simulink model "MX_Gateway"  (Simulink 9.0) (continued)
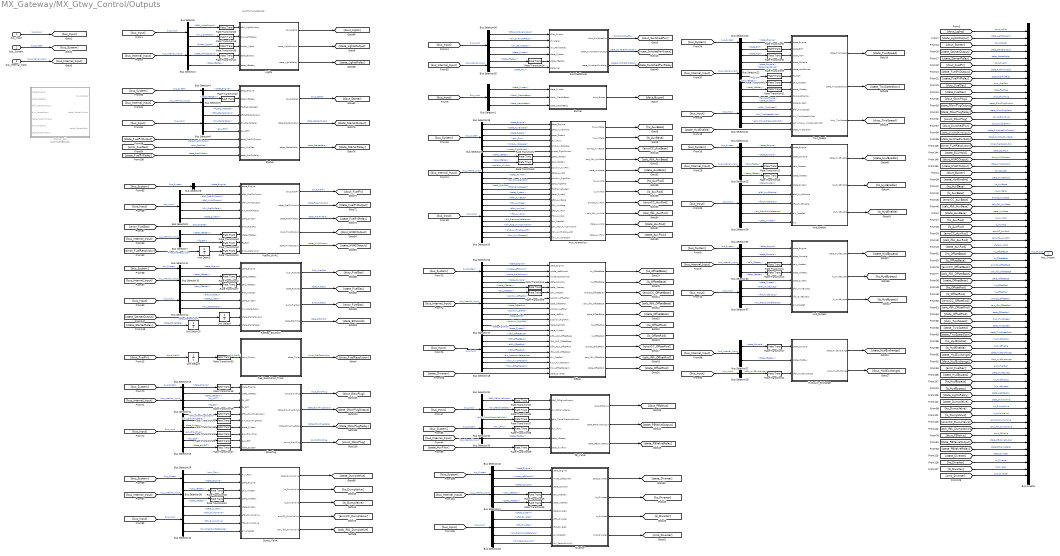
== system MX_Gateway/MX_Gtwy_Control/Outputs ==
  note: OUTPUT HANDLING
  bus_Input : Inport
  bus_System : Inport  Port=2
  bus_Internal_Input : Inport  Port=3
  Aux_Hydraulics : Subsystem  TreatAsAtomicUnit=on  SystemSampleTime=srate  RTWFileName=mod_Outputs
      settings: RTWSystemCode=Nonreusable function  RTWFcnNameOpts=Use subsystem name  RTWFileNameOpts=User specified
  Bus Creator : BusCreator  Inputs=67
  Bus Selector : BusSelector  OutputSignals=bus_DigitalInputs.dfbk_LightsOutput,bus_DigitalInputs.din_LightsRelay
  Bus Selector1 : BusSelector  OutputSignals=bus_Controller.state_System,bus_CAN.bus_Secondary.delay_DepthBeep,bus_CAN.bus_Secondary.state_DepthBeep
  Bus Selector10 : BusSelector  OutputSignals=state_VBattery,calc_VBattery
  Bus Selector{4,8,11,13,23..24,33} (7 alike) : BusSelector  OutputSignals=state_Engine
  Bus Selector12 : BusSelector  OutputSignals=bus_AnalogFdbk.afbk_FuelSec,bus_Controller.state_System,bus_DigitalInputs.din_StarterRelay
  Bus Selector14 : BusSelector  OutputSignals=state_VBattery,flt_HOT,flt_ECT
  Bus Selector15 : BusSelector  OutputSignals=bus_DigitalInputs.dfbk_GlowPlugOutput,bus_DigitalInputs.din_GlowPlugRelay,bus_Controller.state_System,bus_CAN.bus_ECU.ecu_GlowPlug,bus_CAN.bus_ECU.ecu_ECT,bus_CAN.bus_ECU.state_ecuECT
  Bus Selector16 : BusSelector  OutputSignals=state_Engine,mode_LeftControl,mode_RightControl
  Bus Selector17 : BusSelector  OutputSignals=state_Console,state_VBattery,calc_VBattery,position_OffsetPot,state_OffsetPot,position_LeftPot,state_LeftPot,position_RightPot,state_RightPot
  Bus Selector18 : BusSelector  OutputSignals=bus_Controller.state_System,bus_AnalogFdbk.afbk_OffsetBase,bus_CurrentFdbk.ifbk_OffsetBase,bus_AnalogFdbk.afbk_OffsetRod,bus_CurrentFdbk.ifbk_OffsetRod,bus_AnalogInputs.ain_PrecisionReference,bus_CAN.bus_DeluxeG5.flow_Aux2Percent,bus_CAN.bus_DeluxeG5.flow_OffsetPercent
  Bus Selector19 : BusSelector  OutputSignals=state_Engine,state_AuxEnable,state_Detent,aux_Flow,cmd_AuxRelease,mode_RightControl
  Bus Selector2 : BusSelector  OutputSignals=button_Lights,state_PanelButtons,state_VBattery
  Bus Selector20 : BusSelector  OutputSignals=bus_Controller.state_System,bus_AnalogFdbk.afbk_AuxBase,bus_CurrentFdbk.ifbk_AuxBase,bus_AnalogFdbk.afbk_AuxRod,bus_CurrentFdbk.ifbk_AuxRod,bus_AnalogInputs.ain_PrecisionReference,bus_CAN.bus_ACD.can_ACD_AuxCommand,bus_CAN.bus_ACD.cmd_AuxEnable_ACD,bus_CAN.bus_DeluxeG5.flow_Aux1Percent
  Bus Selector21 : BusSelector  OutputSignals=bus_Controller.state_System,bus_AnalogFdbk.afbk_TwoSpeed,bus_DigitalInputs.din_TwoSpeedSwitch,bus_CombinedInputs.adin_TravelPressureSwitch
  Bus Selector22 : BusSelector  OutputSignals=state_ECT,state_HOT,state_Console,state_LoadSense,flt_HOT,calc_VBattery,state_VBattery,bar_LoadSense
  Bus Selector25 : BusSelector  OutputSignals=state_Console,calc_VBattery,state_VBattery
  Bus Selector26 : BusSelector  OutputSignals=bus_Controller.state_System,bus_AnalogFdbk.afbk_HydEnable,bus_CurrentFdbk.ifbk_HydEnable,bus_AnalogInputs.ain_PrecisionReference,CANOpenInMsg.state_Inverter
  Bus Selector27 : BusSelector  OutputSignals=state_Console,state_VBattery
  Bus Selector28 : BusSelector  OutputSignals=bus_DigitalInputs.dfbk_HydExchange
  Bus Selector29 : BusSelector  OutputSignals=aux_Flow,state_Engine
  Bus Selector3 : BusSelector  OutputSignals=state_VBattery,calc_VBattery,position_AuxPot,state_AuxPot,position_RightPot,state_RightPot,flt_EngineSpeed
  Bus Selector30 : BusSelector  OutputSignals=state_VBattery
  Bus Selector31 : BusSelector  OutputSignals=bus_DigitalInputs.dfbk_PBValveOutput,bus_DigitalInputs.din_PBValveRelay,bus_CombinedInputs.adin_TravelPressureSwitch
  Bus Selector32 : BusSelector  OutputSignals=aux_Flow
  Bus Selector34 : BusSelector  OutputSignals=state_Console,calc_VBattery,state_VBattery
  Bus Selector35 : BusSelector  OutputSignals=state_VBattery,state_Key
  Bus Selector36 : BusSelector  OutputSignals=bus_DigitalInputs.dfbk_SwPowerOutput,bus_DigitalInputs.din_SwPowerRelay,bus_DigitalInputs.din_RemoteKey,bus_Controller.state_System
  Bus Selector37 : BusSelector  OutputSignals=bus_Controller.state_System,bus_AnalogFdbk.afbk_HydBypass,bus_CurrentFdbk.ifbk_HydBypass,bus_AnalogInputs.ain_PrecisionReference
  Bus Selector38 : BusSelector  OutputSignals=calc_VBattery,state_VBattery
  Bus Selector42 : BusSelector  OutputSignals=bus_Controller.state_System,bus_AnalogFdbk.afbk_DumpValve,bus_CurrentFdbk.ifbk_DumpValve,bus_AnalogInputs.ain_PrecisionReference
  Bus Selector46 : BusSelector  OutputSignals=state_Engine,mode_LeftControl
  Bus Selector47 : BusSelector  OutputSignals=state_Console,calc_VBattery,state_VBattery
  Bus Selector48 : BusSelector  OutputSignals=bus_Controller.state_System,bus_AnalogFdbk.afbk_Diverter,bus_CurrentFdbk.ifbk_Diverter,bus_AnalogInputs.ain_PrecisionReference,bus_DigitalInputs.din_SecondAuxSw
  Bus Selector5 : BusSelector  OutputSignals=state_VBattery,flt_EngineSpeed
  Bus Selector6 : BusSelector  OutputSignals=bus_DigitalInputs.dfbk_StarterOutput,bus_DigitalInputs.din_StarterRelay,bus_CAN.bus_ECU.ecu_EOT
  Bus Selector7 : BusSelector  OutputSignals=state_VBattery,flt_ECT
  Bus Selector9 : BusSelector  OutputSignals=bus_DigitalInputs.dfbk_FuelPriOutput,bus_DigitalInputs.dfbk_HVACOutput,bus_DigitalInputs.din_FuelPriRelay,bus_Controller.state_System
  Buzzer : Subsystem  TreatAsAtomicUnit=on  SystemSampleTime=srate  RTWFileName=mod_Outputs
      settings: RTWSystemCode=Nonreusable function  RTWFileNameOpts=User specified
  Diverter : Subsystem  TreatAsAtomicUnit=on  SystemSampleTime=srate  RTWFileName=mod_Outputs
      settings: RTWSystemCode=Nonreusable function  RTWFcnNameOpts=Use subsystem name  RTWFileNameOpts=User specified
  Dump_Valve : Subsystem  TreatAsAtomicUnit=on  SystemSampleTime=srate  RTWFileName=mod_Outputs
      settings: RTWSystemCode=Nonreusable function  RTWFcnNameOpts=Use subsystem name  RTWFileNameOpts=User specified
  FuelPri_HVAC : Subsystem  TreatAsAtomicUnit=on  SystemSampleTime=srate  RTWFileName=mod_Outputs
      settings: RTWSystemCode=Nonreusable function  RTWFileNameOpts=User specified
  FuelSec_ECUHold : Subsystem  TreatAsAtomicUnit=on  SystemSampleTime=srate  RTWFileName=mod_Outputs
      settings: RTWSystemCode=Nonreusable function  RTWFileNameOpts=User specified
  Fuel_Resolution_Timer : Subsystem  TreatAsAtomicUnit=on  SystemSampleTime=srate100  RTWFileName=mod_Outputs  mask{CD_PRIMARY_FUNCTION=CD_FUEL_PRIMARY_FUNCTION, CD_RESOLUTION_TIMER=CD_FUEL_RESOLUTION_TIMER}
      mask prompts: CD_PRIMARY_FUNCTION "Primary Function"; CD_RESOLUTION_TIMER "Resolution Timer"
      settings: RTWSystemCode=Nonreusable function  RTWFcnNameOpts=Use subsystem name  RTWFileNameOpts=User specified
  GlowPlug : Subsystem  TreatAsAtomicUnit=on  SystemSampleTime=srate100  RTWFileName=mod_Outputs
      settings: RTWSystemCode=Nonreusable function  RTWFileNameOpts=User specified
  Hyd_Bypass : Subsystem  TreatAsAtomicUnit=on  SystemSampleTime=srate  RTWFileName=mod_Outputs
      settings: RTWSystemCode=Nonreusable function  RTWFcnNameOpts=Use subsystem name  RTWFileNameOpts=User specified
  Hyd_Enable : Subsystem  TreatAsAtomicUnit=on  SystemSampleTime=srate  RTWFileName=mod_Outputs
      settings: RTWSystemCode=Nonreusable function  RTWFcnNameOpts=Use subsystem name  RTWFileNameOpts=User specified
  Hydraulic_Exchange : Subsystem  TreatAsAtomicUnit=on  SystemSampleTime=srate100  RTWFileName=mod_Outputs
      settings: RTWSystemCode=Nonreusable function  RTWFcnNameOpts=Use subsystem name  RTWFileNameOpts=User specified
  Lights : Subsystem  TreatAsAtomicUnit=on  SystemSampleTime=srate100  RTWFileName=mod_Outputs
      settings: RTWSystemCode=Nonreusable function  RTWFileNameOpts=User specified
  Offset : Subsystem  TreatAsAtomicUnit=on  SystemSampleTime=srate  RTWFileName=mod_Outputs
      settings: RTWSystemCode=Nonreusable function  RTWFcnNameOpts=Use subsystem name  RTWFileNameOpts=User specified
  PB_Valve : Subsystem  TreatAsAtomicUnit=on  SystemSampleTime=srate100  RTWFileName=mod_Outputs
      settings: RTWSystemCode=Nonreusable function  RTWFileNameOpts=User specified
  Rate Transition{1..18,20..22,24..42,44..46} (43 alike) : RateTransition  Integrity=off
  Starter : Subsystem  TreatAsAtomicUnit=on  SystemSampleTime=srate  RTWFileName=mod_Outputs
      settings: RTWSystemCode=Nonreusable function  RTWFileNameOpts=User specified
  Starter_old : Subsystem  Commented=on  TreatAsAtomicUnit=on  SystemSampleTime=srate  RTWFileName=mod_Outputs
      settings: RTWSystemCode=Nonreusable function  RTWFileNameOpts=User specified
  SwitchedPower : Subsystem  TreatAsAtomicUnit=on  SystemSampleTime=srate  RTWFileName=mod_Outputs
      settings: RTWSystemCode=Nonreusable function  RTWFileNameOpts=User specified
  Two_Speed : Subsystem  TreatAsAtomicUnit=on  SystemSampleTime=srate  RTWFileName=mod_Outputs
      settings: RTWSystemCode=Nonreusable function  RTWFcnNameOpts=Use subsystem name  RTWFileNameOpts=User specified
  Unit Delay{3,5,9..10} (4 alike) : UnitDelay  SampleTime=-1
      settings: AttributesFormatString=<initial=%<X0>>\n<tsample=%<SampleTime>>
  bus_Output : Outport
  wires:
  Lights/1(dout_Lights) -> [dout_Lights]
  Bus Selector4/1 -> Starter/1(state_Engine)  "<state_Engine>"
  Starter/1(dout_Starter) -> [dout_Starter]  "dout_Starter"
  FuelPri_HVAC/1(dout_FuelPri) -> [dout_FuelPri]  "dout_FuelPri"
  FuelPri_HVAC/3(state_FuelPriRelay) -> [state_FuelPriRelay]  "state_FuelPriRelay"
  FuelPri_HVAC/2(state_FuelPriOutput) -> [state_FuelPriOutput]  "state_FuelPriOutput"
  Starter/2(state_StarterOutput) -> [state_StarterOutput]  "state_StarterOutput"
  Starter/3(state_StarterRelay) -> [state_StarterRelay]  "state_StarterRelay"
  Lights/2(state_LightsOutput) -> [state_LightsOutput]
  [error_FuelSec] -> Starter/8(error_FuelSec)  "error_FuelSec"
  Bus Selector14/3 -> GlowPlug/4(flt_ECT)  "<flt_ECT>"
  Bus Selector14/2 -> GlowPlug/3(flt_HOT)  "<flt_HOT>"
  Bus Selector14/1 -> GlowPlug/2(state_VBattery)  "<state_VBattery>"
  GlowPlug/1(dout_GlowPlug) -> [dout_GlowPlug]  "dout_GlowPlug"
  GlowPlug/2(state_GlowPlugOutput) -> [state_GlowPlugOutput]  "state_GlowPlugOutput"
  GlowPlug/3(state_GlowPlugRelay) -> [state_GlowPlugRelay]  "state_GlowPlugRelay"
  FuelSec_ECUHold/1(duty_FuelSec) -> [duty_FuelSec]  "duty_FuelSec"
  FuelSec_ECUHold/2(state_FuelSec) -> [state_FuelSec]  "state_FuelSec"
  [state_FuelPriRelay] -> Starter/9(state_FuelPriRelay)  "state_FuelPriRelay"
  GlowPlug/4(count_GlowPlug) -> [count_GlowPlug]  "count_GlowPlug"
  Rate Transition16/1 -> GlowPlug/1(state_Engine)
  SwitchedPower/1(dout_SwitchedPwr) -> [dout_SwitchedPwr]  "dout_SwitchedPwr"
  SwitchedPower/2(state_SwitchedPwrOutput) -> [state_SwitchedPwrOutput]  "state_SwitchedPwrOutput"
  SwitchedPower/3(state_SwitchedPwrRelay) -> [state_SwitchedPwrRelay]  "state_SwitchedPwrRelay"
  Bus Selector36/1 -> SwitchedPower/1(dkbk_Output)  "<dfbk_SwPowerOutput>"
  Bus Selector36/2 -> SwitchedPower/2(din_Relay)  "<din_SwPowerRelay>"
  Bus Selector35/1 -> Rate Transition14/1  "<state_VBattery>"
  Bus Selector35/2 -> SwitchedPower/6(state_Key)  "<state_Key>"
  bus_Input/1 -> [bus_Input]  "bus_Input"
  bus_System/1 -> [bus_System]  "bus_System"
  bus_Internal_Input/1 -> [bus_Internal_Input]  "bus_Internal_Input"
  [bus_Input] -> Bus Selector/1  "bus_Input"
  [bus_Internal_Input] -> Bus Selector2/1  "bus_Internal_Inputs"
  [dout_Lights] -> Bus Creator/1  "dout_Lights"
  [state_LightsOutput] -> Bus Creator/2  "state_LightsOutput"
  [dout_Starter] -> Bus Creator/3  "dout_Starter"
  [state_StarterOutput] -> Bus Creator/4  "state_StarterOutput"
  [state_StarterRelay] -> Bus Creator/5  "state_StarterRelay"
  [dout_FuelPri] -> Bus Creator/6  "dout_FuelPri"
  [state_FuelPriOutput] -> Bus Creator/7  "state_FuelPriOutput"
  [state_FuelPriRelay] -> Bus Creator/8  "state_FuelPriRelay"
  [duty_FuelSec] -> Bus Creator/9  "duty_FuelSec"
  [state_FuelSec] -> Bus Creator/10  "state_FuelSec"
  [dout_GlowPlug] -> Bus Creator/11  "dout_GlowPlug"
  [state_GlowPlugOutput] -> Bus Creator/12  "state_GlowPlugOutput"
  [state_GlowPlugRelay] -> Bus Creator/13  "state_GlowPlugRelay"
  [count_GlowPlug] -> Bus Creator/14  "count_GlowPlug"
  [dout_SwitchedPwr] -> Bus Creator/15  "dout_SwitchedPwr"
  [state_SwitchedPwrOutput] -> Bus Creator/16  "state_SwitchedPwrOutput"
  [state_SwitchedPwrRelay] -> Bus Creator/17  "state_SwitchedPwrRelay"
  Bus Selector2/3 -> Lights/5(state_VBattery)  "<state_VBattery>"
  [bus_System] -> Bus Selector4/1  "bus_System"
  [state_FuelPriOutput] -> Starter/7(state_FuelPriOutput)  "state_FuelPriOutput"
  [bus_Internal_Input] -> Bus Selector5/1  "bus_Internal_Inputs"
  Bus Selector5/1 -> Rate Transition20/1  "<state_VBattery>"
  [bus_Input] -> Bus Selector6/1  "bus_Input"
  Bus Selector6/1 -> Starter/4(dfbk_StarterOutput)  "<dfbk_StarterOutput>"
  Bus Selector6/2 -> Starter/5(din_StarterRelay)  "<din_StarterRelay>"
  [bus_Internal_Input] -> Bus Selector7/1  "bus_Internal_Inputs"
  Bus Selector8/1 -> FuelPri_HVAC/1(state_Engine)  "<state_Engine>"
  [bus_System] -> Bus Selector8/1  "bus_System"
  [bus_Input] -> Bus Selector9/1  "bus_Input"
  Bus Selector9/1 -> FuelPri_HVAC/2(dfbk_FuelPriOutput)  "<dfbk_FuelPriOutput>"
  Bus Selector9/3 -> FuelPri_HVAC/4(din_FuelPriRelay)  "<din_FuelPriRelay>"
  Bus Selector7/1 -> Rate Transition34/1  "<state_VBattery>"
  [bus_Internal_Input] -> Bus Selector10/1  "bus_Internal_Inputs"
  Bus Selector11/1 -> FuelSec_ECUHold/1(state_Engine)  "<state_Engine>"
  [bus_System] -> Bus Selector11/1  "bus_System"
  [bus_Input] -> Bus Selector12/1  "bus_Input"
  Bus Selector13/1 -> Rate Transition16/1  "<state_Engine>"
  [bus_System] -> Bus Selector13/1  "bus_System"
  [bus_Internal_Input] -> Bus Selector14/1  "bus_Internal_Input"
  [bus_Input] -> Bus Selector15/1  "bus_Input"
  Bus Selector15/1 -> Rate Transition29/1  "<dfbk_GlowPlugOutput>"
  Bus Selector15/2 -> Rate Transition30/1  "<din_GlowPlugRelay>"
  Bus Selector15/3 -> Rate Transition44/1  "<state_System>"
  [bus_System] -> Bus Selector16/1  "bus_System"
  [bus_Internal_Input] -> Bus Selector17/1  "bus_Internal_Input"
  [bus_Input] -> Bus Selector18/1  "bus_Input"
  [bus_Internal_Input] -> Bus Selector35/1  "bus_Internal_Input"
  [bus_Input] -> Bus Selector36/1  "bus_Input"
  Rate Transition20/1 -> Starter/2(state_VBattery)
  [dout_FuelPri] -> Unit Delay3/1  "dout_FuelPri"
  Unit Delay3/1 -> Rate Transition33/1
  Fuel_Resolution_Timer/1(state_Timer) -> [timer_FuelResolution]  "timer_FuelResolution"
  [timer_FuelResolution] -> Unit Delay5/1  "timer_FuelResolution"
  Unit Delay5/1 -> Rate Transition41/1
  Bus Selector12/2 -> FuelSec_ECUHold/5(state_System)  "<state_System>"
  Bus Selector7/2 -> Rate Transition40/1  "<flt_ECT>"
  Rate Transition33/1 -> Fuel_Resolution_Timer/1(dout_Primary)  "dout_FuelPri"
  Bus Selector/1 -> Rate Transition25/1  "<dfbk_LightsOutput>"
  Bus Selector36/3 -> SwitchedPower/3(din_RemoteKey)  "<din_RemoteKey>"
  FuelSec_ECUHold/3(error_FuelSec) -> [error_FuelSec]  "error_FuelSec"
  [timer_FuelResolution] -> Bus Creator/18  "timer_FuelResolution"
  Rate Transition44/1 -> GlowPlug/7(state_System)
  Rate Transition22/1 -> Lights/4(state_Buttons)
  Rate Transition36/1 -> Lights/3(button_lights)
  Bus Selector10/1 -> Rate Transition42/1  "<state_VBattery>"
  Bus Selector10/2 -> Rate Transition7/1  "<calc_VBattery>"
  Bus Selector12/1 -> FuelSec_ECUHold/4(afbk_FuelSec)  "<afbk_FuelSec>"
  Bus Selector36/4 -> SwitchedPower/4(state_System)  "<state_System>"
  FuelSec_ECUHold/4(state_ECUHold) -> [state_ECUHold]  "state_ECUHold"
  [state_ECUHold] -> Bus Creator/19  "state_ECUHold"
  [state_StarterOutput] -> Unit Delay9/1  "state_StarterOutput"
  [state_StarterRelay] -> Unit Delay10/1  "state_StarterRelay"
  Unit Delay9/1 -> FuelSec_ECUHold/7(state_StarterOutput)
  Unit Delay10/1 -> FuelSec_ECUHold/8(state_StarterRelay)
  Bus Selector12/3 -> FuelSec_ECUHold/6(din_StarterRelay)  "<din_StarterRelay>"
  Rate Transition34/1 -> FuelPri_HVAC/7(state_VBattery)
  Rate Transition40/1 -> FuelPri_HVAC/8(flt_ECT)
  Rate Transition41/1 -> FuelPri_HVAC/9(timer_Resolution)
  Rate Transition42/1 -> FuelSec_ECUHold/2(state_VBattery)
  Rate Transition7/1 -> FuelSec_ECUHold/3(calc_VBattery)
  [error_FuelSec] -> FuelPri_HVAC/6(error_FuelSec)  "error_FuelSec"
  FuelPri_HVAC/4(dout_HVACOutput) -> [dout_HVACOutput]
  FuelPri_HVAC/5(state_HVACOutput) -> [state_HVACOutput]  "state_HVACOutput"
  [dout_HVACOutput] -> Bus Creator/20  "dout_HVACOutput"
  [state_HVACOutput] -> Bus Creator/21  "state_HVACOutput"
  Bus Selector9/4 -> FuelPri_HVAC/5(state_System)  "<state_System>"
  Rate Transition14/1 -> SwitchedPower/5(state_VBattery)
  Buzzer/1(dout_Buzzer) -> [dout_Buzzer]
  [dout_Buzzer] -> Bus Creator/22  "dout_Buzzer"
  [bus_Input] -> Bus Selector1/1  "bus_Input"
  Bus Selector1/1 -> Buzzer/1(state_System)  "<state_System>"
  Two_Speed/1(state_TwoSpeed) -> [state_TwoSpeed]
  [state_HydEnable] -> Bus Creator/23  "state_HydEnable"
  [bus_Internal_Input] -> Bus Selector3/1  "bus_Internal_Input"
  [bus_System] -> Bus Selector19/1  "bus_System"
  [bus_Input] -> Bus Selector20/1  "bus_Input"
  Bus Selector19/1 -> Aux_Hydraulics/1(state_Engine)  "<state_Engine>"
  Bus Selector3/1 -> Rate Transition2/1  "<state_VBattery>"
  Bus Selector3/2 -> Rate Transition3/1  "<calc_VBattery>"
  Bus Selector20/1 -> Aux_Hydraulics/14(state_System)  "<state_System>"
  Bus Selector20/2 -> Aux_Hydraulics/15(afbk_AuxBase)  "<afbk_AuxBase>"
  Bus Selector20/3 -> Aux_Hydraulics/16(ifbk_AuxBase)  "<ifbk_AuxBase>"
  Bus Selector20/4 -> Aux_Hydraulics/17(afbk_AuxRod)  "<afbk_AuxRod>"
  Bus Selector20/5 -> Aux_Hydraulics/18(ifbk_AuxRod)  "<ifbk_AuxRod>"
  Aux_Hydraulics/1(hs_AuxBase) -> [hs_AuxBase]
  Aux_Hydraulics/2(ls_AuxBase) -> [ls_AuxBase]
  Aux_Hydraulics/3(errorCC_AuxBase) -> [errorCC_AuxBase]
  Aux_Hydraulics/4(calc_ifbk_AuxBase) -> [calc_ifbk_AuxBase]
  Aux_Hydraulics/5(state_AuxBase) -> [state_AuxBase]
  Aux_Hydraulics/6(hs_AuxRod) -> [hs_AuxRod]
  Aux_Hydraulics/7(ls_AuxRod) -> [ls_AuxRod]
  Aux_Hydraulics/8(errorCC_AuxRod) -> [errorCC_AuxRod]
  Aux_Hydraulics/9(calc_ifbk_AuxRod) -> [calc_ifbk_AuxRod]
  Aux_Hydraulics/10(state_AuxRod) -> [state_AuxRod]
  [hs_AuxBase] -> Bus Creator/24  "hs_AuxBase"
  [errorCC_AuxBase] -> Bus Creator/26  "errorCC_AuxBase"
  [calc_ifbk_AuxBase] -> Bus Creator/27  "calc_ifbk_AuxBase"
  [state_AuxBase] -> Bus Creator/28  "state_AuxBase"
  [hs_AuxRod] -> Bus Creator/29  "hs_AuxRod"
  [ls_AuxRod] -> Bus Creator/30  "ls_AuxRod"
  [errorCC_AuxRod] -> Bus Creator/31  "errorCC_AuxRod"
  [calc_ifbk_AuxRod] -> Bus Creator/32  "calc_ifbk_AuxRod"
  [state_AuxRod] -> Bus Creator/33  "state_AuxRod"
  Bus Selector16/1 -> Offset/1(state_Engine)  "<state_Engine>"
  Bus Selector18/1 -> Offset/13(state_System)  "<state_System>"
  Bus Selector17/1 -> Offset/4(state_Console)  "<state_Console>"
  Bus Selector17/2 -> Rate Transition4/1  "<state_VBattery>"
  Bus Selector17/3 -> Rate Transition5/1  "<calc_VBattery>"
  Bus Selector17/4 -> Offset/7(position_OffsetPot)  "<position_OffsetPot>"
  Bus Selector17/5 -> Offset/8(state_OffsetPot)  "<state_OffsetPot>"
  Bus Selector18/2 -> Offset/14(afbk_A2D_OffsetBase)  "<afbk_OffsetBase>"
  Bus Selector18/3 -> Offset/15(ifbk_A2D_OffsetBase)  "<ifbk_OffsetBase>"
  Bus Selector18/4 -> Offset/16(afbk_A2D_OffsetRod)  "<afbk_OffsetRod>"
  Bus Selector18/5 -> Offset/17(ifbk_A2D_OffsetRod)  "<ifbk_OffsetRod>"
  Offset/1(hs_OffsetBase) -> [hs_OffsetBase]
  Offset/2(ls_OffsetBase) -> [ls_OffsetBase]
  Offset/3(errorCC_OffsetBase) -> [errorCC_OffsetBase]
  Offset/4(calc_ifbk_OffsetBase) -> [calc_ifbk_OffsetBase]
  Offset/5(state_OffsetBase) -> [state_OffsetBase]
  Offset/6(hs_OffsetRod) -> [hs_OffsetRod]
  Offset/7(ls_OffsetRod) -> [ls_OffsetRod]
  Offset/8(errorCC_OffsetRod) -> [errorCC_OffsetRod]
  Offset/9(calc_ifbk_OffsetRod) -> [calc_ifbk_OffsetRod]
  Offset/10(state_OffsetRod) -> [state_OffsetRod]
  [hs_OffsetBase] -> Bus Creator/34  "hs_OffsetBase"
  [ls_OffsetBase] -> Bus Creator/35  "ls_OffsetBase"
  [errorCC_OffsetBase] -> Bus Creator/36  "errorCC_OffsetBase"
  [calc_ifbk_OffsetBase] -> Bus Creator/37  "calc_ifbk_OffsetBase"
  [state_OffsetBase] -> Bus Creator/38  "state_OffsetBase"
  [hs_OffsetRod] -> Bus Creator/39  "hs_OffsetRod"
  [ls_OffsetRod] -> Bus Creator/40  "ls_OffsetRod"
  [errorCC_OffsetRod] -> Bus Creator/41  "errorCC_OffsetRod"
  [calc_ifbk_OffsetRod] -> Bus Creator/42  "calc_ifbk_OffsetRod"
  [state_OffsetRod] -> Bus Creator/43  "state_OffsetRod"
  Two_Speed/2(state_TwoSpeedIcon) -> [state_TwoSpeedIcon]
  Two_Speed/3(duty_TwoSpeed) -> [duty_TwoSpeed]
  [bus_Input] -> Bus Selector21/1  "bus_Input"
  Bus Selector21/1 -> Two_Speed/10(state_System)  "<state_System>"
  Bus Selector21/2 -> Two_Speed/11(afbk_TwoSpeed)  "<afbk_TwoSpeed>"
  Bus Selector21/3 -> Two_Speed/12(din_TwoSpeedSwitch)  "<din_TwoSpeedSwitch>"
  Bus Selector21/4 -> Two_Speed/13(adin_TravelPressureSwitch)  "<adin_TravelPressureSwitch>"
  [bus_Internal_Input] -> Bus Selector22/1  "bus_Internal_Input"
  Bus Selector22/1 -> Rate Transition6/1  "<state_ECT>"
  Bus Selector22/2 -> Rate Transition8/1  "<state_HOT>"
  Bus Selector22/3 -> Two_Speed/4(state_Console)  "<state_Console>"
  Bus Selector22/4 -> Two_Speed/5(state_LoadSense)  "<state_LoadSense>"
  Bus Selector22/5 -> Rate Transition9/1  "<flt_HOT>"
  Bus Selector22/6 -> Rate Transition10/1  "<calc_VBattery>"
  Bus Selector22/7 -> Rate Transition11/1  "<state_VBattery>"
  [bus_System] -> Bus Selector23/1  "bus_System"
  Bus Selector23/1 -> Two_Speed/1(state_Engine)  "<state_Engine>"
  Hyd_Enable/1(state_HydEnable) -> [state_HydEnable]
  [state_HydEnable] -> Two_Speed/14(state_HydEnable)
  [bus_Internal_Input] -> Bus Selector25/1  "bus_Internal_Input"
  [bus_Input] -> Bus Selector26/1  "bus_Input"
  Bus Selector25/1 -> Hyd_Enable/2(state_Console)  "<state_Console>"
  Bus Selector25/2 -> Rate Transition13/1  "<calc_VBattery>"
  Bus Selector25/3 -> Rate Transition15/1  "<state_VBattery>"
  Bus Selector26/1 -> Hyd_Enable/5(state_System)  "<state_System>"
  Bus Selector26/2 -> Hyd_Enable/6(afbk_HydEnable)  "<afbk_HydEnable>"
  Bus Selector26/3 -> Hyd_Enable/7(ifbk_HydEnable)  "<ifbk_HydEnable>"
  Hyd_Enable/2(hs_HydEnable) -> [hs_HydEnable]
  Hyd_Enable/3(ls_HydEnable) -> [ls_HydEnable]
  [duty_TwoSpeed] -> Bus Creator/44  "duty_TwoSpeed"
  [state_TwoSpeed] -> Bus Creator/45  "state_TwoSpeed"
  [state_TwoSpeedIcon] -> Bus Creator/46  "state_TwoSpeedIcon"
  [hs_HydEnable] -> Bus Creator/47  "hs_HydEnable"
  [ls_HydEnable] -> Bus Creator/48  "ls_HydEnable"
  [bus_Internal_Input] -> Bus Selector27/1  "bus_Internal_Input"
  Bus Selector27/1 -> Rate Transition17/1  "<state_Console>"
  Bus Selector27/2 -> Hydraulic_Exchange/2(state_Vbattery)  "<state_VBattery>"
  [bus_Input] -> Bus Selector28/1  "bus_Input"
  Bus Selector28/1 -> Rate Transition21/1  "<dfbk_HydExchange>"
  Hydraulic_Exchange/1(state_HydExchange) -> [state_HydExchange]
  Hydraulic_Exchange/2(dout_HydExchange) -> [dout_HydExchange]
  [state_HydExchange] -> Bus Creator/49  "state_HydExchange"
  [dout_HydExchange] -> Bus Creator/50  "dout_HydExchange"
  [error_FuelSec] -> Bus Creator/51  "error_FuelSec"
  Rate Transition6/1 -> Two_Speed/2(state_ECT)
  Rate Transition8/1 -> Two_Speed/3(state_HOT)
  Rate Transition9/1 -> Two_Speed/6(flt_HOT)
  Rate Transition10/1 -> Two_Speed/7(calc_Vbattery)
  Rate Transition11/1 -> Two_Speed/8(state_Vbattery)
  Rate Transition13/1 -> Hyd_Enable/3(calc_Vbattery)
  Rate Transition15/1 -> Hyd_Enable/4(state_Vbattery)
  Rate Transition17/1 -> Hydraulic_Exchange/1(state_Console)
  Bus Selector22/8 -> Two_Speed/9(bar_LoadSense)  "<bar_LoadSense>"
  Bus Selector19/2 -> Aux_Hydraulics/2(state_AuxEnable)  "<state_AuxEnable>"
  Bus Selector19/3 -> Aux_Hydraulics/3(state_Detent)  "<state_Detent>"
  Bus Selector19/4 -> Aux_Hydraulics/4(aux_Flow)  "<aux_Flow>"
  Bus Selector3/3 -> Aux_Hydraulics/9(position_AuxPot)  "<position_AuxPot>"
  Bus Selector3/4 -> Aux_Hydraulics/10(state_AuxPot)  "<state_AuxPot>"
  Rate Transition2/1 -> Aux_Hydraulics/7(state_Vbattery)
  Rate Transition3/1 -> Aux_Hydraulics/8(calc_Vbattery)
  Rate Transition4/1 -> Offset/5(state_Vbattery)
  Rate Transition5/1 -> Offset/6(calc_Vbattery)
  Bus Selector20/6 -> Aux_Hydraulics/19(ain_PrecRef)  "<ain_PrecisionReference>"
  Bus Selector18/6 -> Offset/18(ain_PrecRef)  "<ain_PrecisionReference>"
  Bus Selector26/4 -> Hyd_Enable/8(ain_PrecRef)  "<ain_PrecisionReference>"
  Rate Transition25/1 -> Lights/1(dfbk_LightsOutput)
  Rate Transition29/1 -> GlowPlug/5(dfbk_GlowPlugOutput)
  Rate Transition30/1 -> GlowPlug/6(din_GlowPlugRelay)
  Bus Selector9/2 -> FuelPri_HVAC/3(dfbk_HVACOutput)  "<dfbk_HVACOutput>"
  Rate Transition21/1 -> Hydraulic_Exchange/3(dfbk_HydExchange)
  [ls_AuxBase] -> Bus Creator/25  "ls_AuxBase"
  Bus Selector2/1 -> Rate Transition36/1  "<button_Lights>"
  Bus Selector2/2 -> Rate Transition22/1  "<state_PanelButtons>"
  Hyd_Bypass/1(state_HydBypass) -> [state_HydBypass]
  [bus_System] -> Bus Selector33/1  "bus_System"
  Bus Selector33/1 -> Hyd_Bypass/1(state_Engine)  "<state_Engine>"
  [bus_Internal_Input] -> Bus Selector34/1  "bus_Internal_Input"
  [bus_Input] -> Bus Selector37/1  "bus_Input"
  Bus Selector34/1 -> Hyd_Bypass/2(state_Console)  "<state_Console>"
  Bus Selector34/2 -> Rate Transition1/1  "<calc_VBattery>"
  Bus Selector34/3 -> Rate Transition12/1  "<state_VBattery>"
  Bus Selector37/1 -> Hyd_Bypass/5(state_System)  "<state_System>"
  Bus Selector37/2 -> Hyd_Bypass/6(afbk_HydBypass)  "<afbk_HydBypass>"
  Bus Selector37/3 -> Hyd_Bypass/7(ifbk_HydBypass)  "<ifbk_HydBypass>"
  Hyd_Bypass/2(hs_HydBypass) -> [hs_HydBypass]
  Hyd_Bypass/3(ls_HydBypass) -> [ls_HydBypass]
  Rate Transition1/1 -> Hyd_Bypass/3(calc_Vbattery)
  Rate Transition12/1 -> Hyd_Bypass/4(state_Vbattery)
  Bus Selector37/4 -> Hyd_Bypass/8(ain_PrecRef)  "<ain_PrecisionReference>"
  [hs_HydBypass] -> Bus Creator/53  "hs_HydBypass"
  [ls_HydBypass] -> Bus Creator/54  "ls_HydBypass"
  [state_HydBypass] -> Bus Creator/52  "state_HydBypass"
  Lights/3(state_LightsRelay) -> [state_LightsRelay]
  Bus Selector/2 -> Rate Transition18/1  "<din_LightsRelay>"
  Rate Transition18/1 -> Lights/2(din_LightsRelay)
  [state_LightsRelay] -> Bus Creator/55  "state_LightsRelay"
  Bus Selector20/7 -> Aux_Hydraulics/20(can_ACD_AuxCommand)  "<can_ACD_AuxCommand>"
  Bus Selector20/8 -> Aux_Hydraulics/21(can_AuxEnable_ACD)  "<cmd_AuxEnable_ACD>"
  Bus Selector19/5 -> Aux_Hydraulics/5(cmd_AuxRelease)  "<cmd_AuxRelease>"
  Bus Selector15/4 -> Rate Transition24/1  "<ecu_GlowPlug>"
  Rate Transition24/1 -> GlowPlug/8(ecu_GlowPlug)
  Bus Creator/1 -> bus_Output/1  "bus_Output"
  Rate Transition26/1 -> GlowPlug/9(ecu_ECT)
  Bus Selector15/5 -> Rate Transition26/1  "<ecu_ECT>"
  Bus Selector15/6 -> Rate Transition27/1  "<state_ecuECT>"
  Rate Transition27/1 -> GlowPlug/10(state_ecuECT)
  [bus_System] -> Bus Selector29/1  "bus_System"
  Bus Selector29/1 -> Dump_Valve/1(aux_Flow)  "<aux_Flow>"
  [bus_Internal_Input] -> Bus Selector38/1  "bus_Internal_Input"
  [bus_Input] -> Bus Selector42/1  "bus_Input"
  Bus Selector38/1 -> Rate Transition28/1  "<calc_VBattery>"
  Bus Selector38/2 -> Rate Transition31/1  "<state_VBattery>"
  Bus Selector42/1 -> Dump_Valve/5(state_System)  "<state_System>"
  Bus Selector42/2 -> Dump_Valve/6(afbk_DumpValve)  "<afbk_DumpValve>"
  Bus Selector42/3 -> Dump_Valve/7(ifbk_DumpValve)  "<ifbk_DumpValve>"
  Rate Transition28/1 -> Dump_Valve/3(calc_Vbattery)
  Rate Transition31/1 -> Dump_Valve/4(state_Vbattery)
  Bus Selector42/4 -> Dump_Valve/8(ain_PrecRef)  "<ain_PrecisionReference>"
  Dump_Valve/1(state_DumpValve) -> [state_DumpValve]
  Dump_Valve/2(hs_DumpValve) -> [hs_DumpValve]
  Dump_Valve/3(ls_DumpValve) -> [ls_DumpValve]
  [hs_DumpValve] -> Bus Creator/57  "hs_DumpValve"
  [ls_DumpValve] -> Bus Creator/58  "ls_DumpValve"
  [state_DumpValve] -> Bus Creator/56  "state_DumpValve"
  Bus Selector29/2 -> Dump_Valve/2(state_Engine)  "<state_Engine>"
  Dump_Valve/4(errorCC_DumpValve) -> [errorCC_DumpValve]
  Dump_Valve/5(calc_ifbk_DumpValve) -> [calc_ifbk_DumpValve]
  [errorCC_DumpValve] -> Bus Creator/59  "errorCC_DumpValve"
  [calc_ifbk_DumpValve] -> Bus Creator/60  "calc_ifbk_DumpValve"
  Bus Selector1/2 -> Buzzer/2(delay_DepthBeep)  "<delay_DepthBeep>"
  Bus Selector1/3 -> Buzzer/3(state_DepthBeep)  "<state_DepthBeep>"
  [bus_Internal_Input] -> Bus Selector30/1  "bus_Internal_Input"
  Bus Selector30/1 -> PB_Valve/5(state_VBattery)  "<state_VBattery>"
  [bus_Input] -> Bus Selector31/1  "bus_Input"
  Bus Selector31/1 -> Rate Transition32/1  "<dfbk_PBValveOutput>"
  Bus Selector31/2 -> Rate Transition35/1  "<din_PBValveRelay>"
  Bus Selector31/3 -> Rate Transition37/1  "<adin_TravelPressureSwitch>"
  [bus_System] -> Bus Selector32/1  "bus_System"
  Bus Selector32/1 -> Rate Transition38/1  "<aux_Flow>"
  PB_Valve/1(dout_PBValve) -> [dout_PBValve]
  PB_Valve/2(state_PBValveOutput) -> [state_PBValveOutput]
  PB_Valve/3(state_PBValveRelay) -> [state_PBValveRelay]
  [dout_PBValve] -> Bus Creator/61  "dout_PBValve"
  [state_PBValveOutput] -> Bus Creator/62  "state_PBValveOutput"
  [state_PBValveRelay] -> Bus Creator/63  "state_PBValveRelay"
  Rate Transition32/1 -> PB_Valve/1(dfbk_PBValveOutput)
  Rate Transition35/1 -> PB_Valve/2(din_PBValveRelay)
  Rate Transition37/1 -> PB_Valve/3(adin_TravelPressureSwitch)
  Rate Transition38/1 -> PB_Valve/4(aux_Flow)
  Aux_Hydraulics/11(state_AuxFlow) -> [state_AuxFlow]
  [state_AuxFlow] -> Rate Transition39/1
  Rate Transition39/1 -> PB_Valve/6(state_AuxFlow)
  Diverter/1(state_Diverter) -> [state_Diverter]
  [bus_System] -> Bus Selector46/1  "bus_System"
  Bus Selector46/1 -> Diverter/1(state_Engine)  "<state_Engine>"
  [bus_Internal_Input] -> Bus Selector47/1  "bus_Internal_Input"
  [bus_Input] -> Bus Selector48/1  "bus_Input"
  Bus Selector47/1 -> Diverter/3(state_Console)  "<state_Console>"
  Bus Selector47/2 -> Rate Transition45/1  "<calc_VBattery>"
  Bus Selector47/3 -> Rate Transition46/1  "<state_VBattery>"
  Bus Selector48/1 -> Diverter/6(state_System)  "<state_System>"
  Bus Selector48/2 -> Diverter/7(afbk_Diverter)  "<afbk_Diverter>"
  Bus Selector48/3 -> Diverter/8(ifbk_Diverter)  "<ifbk_Diverter>"
  Diverter/2(hs_Diverter) -> [hs_Diverter]
  Diverter/3(ls_Diverter) -> [ls_Diverter]
  Rate Transition45/1 -> Diverter/4(calc_Vbattery)
  Rate Transition46/1 -> Diverter/5(state_Vbattery)
  Bus Selector48/4 -> Diverter/9(ain_PrecRef)  "<ain_PrecisionReference>"
  [hs_Diverter] -> Bus Creator/65  "hs_Diverter"
  [ls_Diverter] -> Bus Creator/66  "ls_Diverter"
  [state_Diverter] -> Bus Creator/64  "state_Diverter"
  Bus Selector48/5 -> Diverter/10(din_SecondAuxSw)  "<din_SecondAuxSw>"
  Diverter/4(cmd_Diverter) -> [cmd_Diverter]
  [cmd_Diverter] -> Bus Creator/67  "cmd_Diverter"
  Bus Selector5/2 -> Starter/3(flt_EngineSpeed)  "<flt_EngineSpeed>"
  Bus Selector6/3 -> Starter/6(ecu_EOT)  "<ecu_EOT>"
  Bus Selector3/7 -> Aux_Hydraulics/13(flt_EngineSpeed)  "<flt_EngineSpeed>"
  Bus Selector46/2 -> Diverter/2(mode_LeftControl)  "<mode_LeftControl>"
  Bus Selector16/2 -> Offset/2(mode_LeftControl)  "<mode_LeftControl>"
  Bus Selector3/5 -> Aux_Hydraulics/11(position_RightPot)  "<position_RightPot>"
  Bus Selector3/6 -> Aux_Hydraulics/12(state_RightPot)  "<state_RightPot>"
  Bus Selector16/3 -> Offset/3(mode_RightControl)  "<mode_RightControl>"
  Bus Selector17/6 -> Offset/9(position_LeftPot)  "<position_LeftPot>"
  Bus Selector17/7 -> Offset/10(state_LeftPot)  "<state_LeftPot>"
  Bus Selector17/8 -> Offset/11(position_RightPot)  "<position_RightPot>"
  Bus Selector17/9 -> Offset/12(state_RightPot)  "<state_RightPot>"
  Bus Selector19/6 -> Aux_Hydraulics/6(mode_RightControl)  "<mode_RightControl>"
  [state_Diverter] -> Offset/21(state_Diverter)
  Bus Selector18/7 -> Offset/19(flow_Aux2Percent)  "<flow_Aux2Percent>"
  Bus Selector18/8 -> Offset/20(flow_OffsetPercent)  "<flow_OffsetPercent>"
  Bus Selector20/9 -> Aux_Hydraulics/22(flow_Aux1Percent)  "<flow_Aux1Percent>"
  Bus Selector26/5 -> Hyd_Enable/9(In1)  "<state_Inverter>"
  [bus_System] -> Bus Selector24/1  "bus_System"
  Bus Selector24/1 -> Hyd_Enable/1(state_Engine)  "<state_Engine>"
